simulink model "HvacCtrlrRe"  (R2022b)
settings: Solver=FixedStepDiscrete  StartTime=0.0  StopTime=10.0  FixedStep=0.2  RelTol=1e-3
settings Model: Creator=blue.ge  LastModifiedBy=blue.ge
settings DataIOCC: SaveFormat=StructureWithTime
settings OptimizationCC: DefaultParameterBehavior=Inlined  EnhancedBackFolding=on  PassReuseOutputArgsAs=Individual arguments  ZeroExternalMemoryAtStartup=off  ZeroInternalMemoryAtStartup=off  OptimizeBlockOrder=speed  DifferentSizesBufferReuse=on  OptimizationCustomize=off  EfficientTunableParamExpr=on
settings DebuggingCC: ParameterTunabilityLossMsg=error
settings ModelReferenceCC: ModelReferenceNumInstancesAllowed=Single
settings SFSimCC: MATLABDynamicMemAlloc=off
settings RTWCC: Description=AUTOSAR  SystemTargetFile=autosar.tlc  GenCodeOnly=on  TemplateMakefile=ert_default_tmf
settings CodeAppCC: ForceParamTrailComments=on  IgnoreCustomStorageClasses=off  OperatorAnnotations=on  SimulinkDataObjDesc=on  SFDataObjDesc=on  InsertBlockDesc=on
settings STFCustomTargetCC: TargetLangStandard=C99 (ISO)  MultiwordLength=256  ParMdlRefBuildCompliant=on  CompOptLevelCompliant=on  IncludeMdlTerminateFcn=off  CombineOutputUpdateFcns=on  SuppressErrorStatus=on  ERTCustomFileBanners=on  SupportNonFinite=off  SupportComplex=off  SupportContinuousTime=off  SupportNonInlinedSFcns=off  RemoveResetFunc=on  MATLABClassNameForMDSCustomization=Simulink.SoftwareTarget.ERTCustomization  AutosarCompliant=on  UseToolchainInfoCompliant=on  LookupTableObjectStructAxisOrder=2,1,3,4,...  LUTObjectStructOrderEvenSpacing=Size,Table,Breakpoints  SystemTargetFile=autosar.tlc
settings ERTTargetCC: TargetLangStandard=C99 (ISO)  ConcurrentExecutionCompliant=off  IncludeMdlTerminateFcn=off  SuppressErrorStatus=on  SupportNonFinite=off  SupportComplex=off  ModelStepFunctionPrototypeControlCompliant=off  CPPClassGenCompliant=off  AutosarCompliant=on  LookupTableObjectStructAxisOrder=2,1,3,4,...  LUTObjectStructOrderEvenSpacing=Size,Table,Breakpoints  InlinedParameterPlacement=NonHierarchical  ERTCustomFileTemplate=example_file_process.tlc

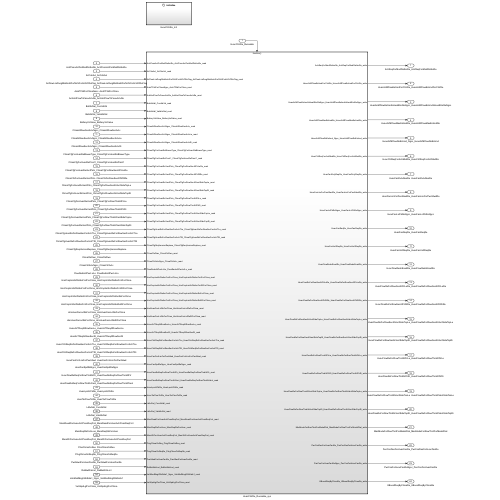
[thumbnail: Simulink's stored preview of the top-level diagram]
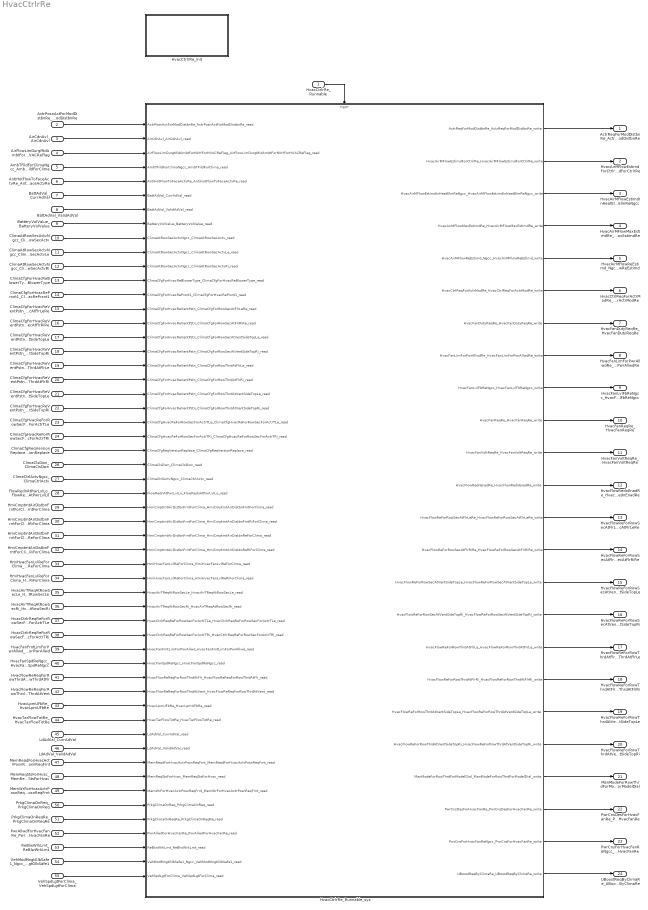
== system HvacCtrlrRe ==
  HvacCtrlrRe_Runnable : Inport  SampleTime=0.2  OutputFunctionCall=on
  ActrPosnActForModDistbnRe_ActrPosnActForModDistbnRe : Inport  Port=2  OutMin=0  OutMax=100  OutDataTypeStr=Perc9  PortDimensions=1
  AirCdnAvl_AirCdnAvl : Inport  Port=3  OutDataTypeStr=Boolean  PortDimensions=1
  AirFlowLimDurgMidAmbtForNVHForHVACReFlag_AirFlowLimDurgMidAmbtForNVHForHVACReFlag : Inport  Port=4  OutDataTypeStr=Boolean  PortDimensions=1
  AmbTFildForClimaNgcc_AmbTFildForClima : Inport  Port=5  OutDataTypeStr=T30  PortDimensions=1
  AntiHotFlowToFaceActvRe_AntiHotFlowToFaceActvRe : Inport  Port=6  OutDataTypeStr=Boolean  PortDimensions=1
  BattAdVal_CurrAdVal : Inport  Port=7  OutDataTypeStr=BatCurrAd  PortDimensions=1
  BattAdVal_ValidAdVal : Inport  Port=8  OutDataTypeStr=BatValidAd  PortDimensions=1
  BatteryVolValue_BatteryVolValue : Inport  Port=9  OutDataTypeStr=BatteryVolValue  PortDimensions=1
  ClimaAtRowSecActvNgcc_ClimaAtRowSecActv : Inport  Port=10  OutDataTypeStr=Boolean  PortDimensions=1
  ClimaAtRowSecActvNgcc_ClimaAtRowSecActvLe : Inport  Port=11  OutDataTypeStr=Boolean  PortDimensions=1
  ClimaAtRowSecActvNgcc_ClimaAtRowSecActvRi : Inport  Port=12  OutDataTypeStr=Boolean  PortDimensions=1
  ClimaCfgForHvacReBlowerType_ClimaCfgForHvacReBlowerType : Inport  Port=13  OutDataTypeStr=Enum: ClimaCfgForHvacReBlowerType  PortDimensions=1
  ClimaCfgForHvacRePrsnt1_ClimaCfgForHvacRePrsnt1 : Inport  Port=14  OutDataTypeStr=Enum: CarCfgForHvacRe  PortDimensions=1
  ClimaCfgForHvacReVentPstn_ClimaCfgForRowSecAtFlrLeRe : Inport  Port=15  OutDataTypeStr=UInt8  PortDimensions=1
  ClimaCfgForHvacReVentPstn_ClimaCfgForRowSecAtFlrRiRe : Inport  Port=16  OutDataTypeStr=UInt8  PortDimensions=1
  ClimaCfgForHvacReVentPstn_ClimaCfgForRowSecAtVentSideTopLe : Inport  Port=17  OutDataTypeStr=UInt8  PortDimensions=1
  ClimaCfgForHvacReVentPstn_ClimaCfgForRowSecAtVentSideTopRi : Inport  Port=18  OutDataTypeStr=UInt8  PortDimensions=1
  ClimaCfgForHvacReVentPstn_ClimaCfgForRowThrdAtFlrLe : Inport  Port=19  OutDataTypeStr=UInt8  PortDimensions=1
  ClimaCfgForHvacReVentPstn_ClimaCfgForRowThrdAtFlrRi : Inport  Port=20  OutDataTypeStr=UInt8  PortDimensions=1
  ClimaCfgForHvacReVentPstn_ClimaCfgForRowThrdAtVentSideTopLe : Inport  Port=21  OutDataTypeStr=UInt8  PortDimensions=1
  ClimaCfgForHvacReVentPstn_ClimaCfgForRowThrdAtVentSideTopRi : Inport  Port=22  OutDataTypeStr=UInt8  PortDimensions=1
  ClimaCfgHvacReForRowSecForActrTLe_ClimaCfgHvacReForRowSecForActrTLe : Inport  Port=23  OutDataTypeStr=UInt8  PortDimensions=1
  ClimaCfgHvacReForRowSecForActrTRi_ClimaCfgHvacReForRowSecForActrTRi : Inport  Port=24  OutDataTypeStr=UInt8  PortDimensions=1
  ClimaCfgReqVersionReplace_ClimaCfgReqVersionReplace : Inport  Port=25  OutDataTypeStr=UInt8  PortDimensions=1
  ClimaClsDwn_ClimaClsDwn : Inport  Port=26  OutDataTypeStr=Boolean  PortDimensions=1
  ClimaCtrlActvNgcc_ClimaCtrlActv : Inport  Port=27  OutDataTypeStr=Boolean  PortDimensions=1
  FlowRednAtPwrLvlLo_FlowRednAtPwrLvlLo : Inport  Port=28  OutDataTypeStr=Boolean  PortDimensions=1
  HmiCmptmtAirDistbnFrntForClima_HmiCmptmtAirDistbnFrntForClima : Inport  Port=29  OutDataTypeStr=Enum: HmiCmptmtAirDistbnFrnt  PortDimensions=1
  HmiCmptmtAirDistbnFrntForClima_HmiCmptmtAirDistbnFrntRiForClima : Inport  Port=30  OutDataTypeStr=Enum: HmiCmptmtAirDistbnFrnt  PortDimensions=1
  HmiCmptmtAirDistbnFrntForClima_HmiCmptmtAirDistbnReForClima : Inport  Port=31  OutDataTypeStr=Enum: HmiCmptmtAirDistbnFrnt  PortDimensions=1
  HmiCmptmtAirDistbnFrntForClima_HmiCmptmtAirDistbnReRiForClima : Inport  Port=32  OutDataTypeStr=Enum: HmiCmptmtAirDistbnFrnt  PortDimensions=1
  HmiHvacFanLvlReForClima_HmiHvacFanLvlReForClima : Inport  Port=33  OutDataTypeStr=Enum: HmiHvacFanLvl  PortDimensions=1
  HmiHvacFanLvlReForClima_HmiHvacFanLvlReRiForClima : Inport  Port=34  OutDataTypeStr=Enum: HmiHvacFanLvl  PortDimensions=1
  HvacAirTReqAtRowSecLe_HvacAirTReqAtRowSecLe : Inport  Port=35  OutDataTypeStr=T30  PortDimensions=1
  HvacAirTReqAtRowSecRi_HvacAirTReqAtRowSecRi : Inport  Port=36  OutDataTypeStr=T30  PortDimensions=1
  HvacCtrlrReqReForRowSecForActrTLe_HvacCtrlrReqReForRowSecForActrTLe : Inport  Port=37  OutDataTypeStr=Perc30  PortDimensions=1
  HvacCtrlrReqReForRowSecForActrTRi_HvacCtrlrReqReForRowSecForActrTRi : Inport  Port=38  OutDataTypeStr=Perc30  PortDimensions=1
  HvacFanFrntLimForPwrAllwd_HvacFanFrntLimForPwrAllwd : Inport  Port=39  OutDataTypeStr=Enum: HvacFanFrntLimForPwrAllwd  PortDimensions=1
  HvacFanSpdReNgcc_HvacFanSpdReNgcc : Inport  Port=40  OutDataTypeStr=HvacFanSpd  PortDimensions=1
  HvacFlowReReqForRowThrdAtFlr_HvacFlowReReqForRowThrdAtFlr : Inport  Port=41  OutDataTypeStr=AirFlow30  PortDimensions=1
  HvacFlowReReqForRowThrdAtVent_HvacFlowReReqForRowThrdAtVent : Inport  Port=42  OutDataTypeStr=AirFlow30  PortDimensions=1
  HvacLpmUFbRe_HvacLpmUFbRe : Inport  Port=43  OutMin=0  OutMax=100  OutDataTypeStr=Perc9  PortDimensions=1
  HvacTarFlowTotRe_HvacTarFlowTotRe : Inport  Port=44  OutDataTypeStr=AirFlow30  PortDimensions=1
  LdAdVal_CurrAdVal : Inport  Port=45  OutDataTypeStr=Bus: LdAd  PortDimensions=1  BusOutputAsStruct=on
  LdAdVal_ValidAdVal : Inport  Port=46  OutDataTypeStr=Bus: LdAd  PortDimensions=1  BusOutputAsStruct=on
  MemReadForHvacActrPosnReqFrnt_MemReadForHvacActrPosnReqFrnt : Inport  Port=47  OutDataTypeStr=Bus: MemRecForHvacActrPosnReqFrnt  PortDimensions=1  BusOutputAsStruct=on
  MemReqStsForHvac_MemReqStsForHvac : Inport  Port=48  OutDataTypeStr=Enum: MemReqSts  PortDimensions=1
  MemWrForHvacActrPosnReqFrnt_MemWrForHvacActrPosnReqFrnt : Inport  Port=49  OutDataTypeStr=Bus: MemRecForHvacActrPosnReqFrnt  PortDimensions=1  BusOutputAsStruct=on
  PrkgClimaOnReq_PrkgClimaOnReq : Inport  Port=50  OutDataTypeStr=Boolean  PortDimensions=1
  PrkgClimaOnReqRe_PrkgClimaOnReqRe : Inport  Port=51  OutDataTypeStr=Enum: OnOff1  PortDimensions=1
  PwrAllwdForHvacFanRe_PwrAllwdForHvacFanRe : Inport  Port=52  OutDataTypeStr=Pwr33  PortDimensions=1
  ReBlwWrkLmt_ReBlwWrkLmt : Inport  Port=53  OutDataTypeStr=UInt8  PortDimensions=1
  VehModMngtGlbSafe1_Ngcc_VehModMngtGlbSafe1 : Inport  Port=54  OutDataTypeStr=Bus: VehModMngtGlbSafe1  PortDimensions=1  BusOutputAsStruct=on
  VehSpdLgtForClima_VehSpdLgtForClima : Inport  Port=55  OutDataTypeStr=Bus: VehSpdLgtForClima  PortDimensions=1  BusOutputAsStruct=on
  HvacCtrlrRe_Init : Subsystem
  HvacCtrlrRe_Runnable_sys : Subsystem
  ActrReqForModDistbnRe_ActrReqForModDistbnRe : Outport  OutMin=0  OutMax=100  OutDataTypeStr=Perc9  PortDimensions=1
  HvacAirMFlowEstimdForCtrlrRe_HvacAirMFlowEstimdForCtrlrRe : Outport  Port=2  OutDataTypeStr=AirFlow30  PortDimensions=1
  HvacAirMFlowEstimdInHeatElmReNgcc_HvacAirMFlowEstimdInHeatElmReNgcc : Outport  Port=3  OutDataTypeStr=AirFlow30  PortDimensions=1
  HvacAirMFlowMaxEstimdRe_HvacAirMFlowMaxEstimdRe : Outport  Port=4  OutDataTypeStr=AirFlow30  PortDimensions=1
  HvacAirMFlowReEstimd_Ngcc_HvacAirMFlowReEstimd : Outport  Port=5  OutMin=0  OutMax=1000  OutDataTypeStr=MFlow1  PortDimensions=1
  HvacCtrlReqForActrModRe_HvacCtrlReqForActrModRe : Outport  Port=6  OutDataTypeStr=Perc30  PortDimensions=1
  HvacFanDutyReqRe_HvacFanDutyReqRe : Outport  Port=7  OutMin=0  OutMax=100  OutDataTypeStr=Perc9  PortDimensions=1
  HvacFanLimForPwrAllwdRe_HvacFanLimForPwrAllwdRe : Outport  Port=8  OutDataTypeStr=Enum: HvacFanFrntLimForPwrAllwd  PortDimensions=1
  HvacFanLvlFbReNgcc_HvacFanLvlFbReNgcc : Outport  Port=9  OutDataTypeStr=Enum: HmiHvacFanLvl  PortDimensions=1
  HvacFanReqRe_HvacFanReqRe : Outport  Port=10  OutDataTypeStr=HvacFanSpd  PortDimensions=1
  HvacFanVoltReqRe_HvacFanVoltReqRe : Outport  Port=11  OutMin=0  OutMax=100  OutDataTypeStr=Perc9  PortDimensions=1
  HvacFlowRednEnadRe_HvacFlowRednEnadRe : Outport  Port=12  OutDataTypeStr=Boolean  PortDimensions=1
  HvacFlowReForRowSecAtFlrLeRe_HvacFlowReForRowSecAtFlrLeRe : Outport  Port=13  OutDataTypeStr=AirFlow30  PortDimensions=1
  HvacFlowReForRowSecAtFlrRiRe_HvacFlowReForRowSecAtFlrRiRe : Outport  Port=14  OutDataTypeStr=AirFlow30  PortDimensions=1
  HvacFlowReForRowSecAtVentSideTopLe_HvacFlowReForRowSecAtVentSideTopLe : Outport  Port=15  OutDataTypeStr=AirFlow30  PortDimensions=1
  HvacFlowReForRowSecAtVentSideTopRi_HvacFlowReForRowSecAtVentSideTopRi : Outport  Port=16  OutDataTypeStr=AirFlow30  PortDimensions=1
  HvacFlowReForRowThrdAtFlrLe_HvacFlowReForRowThrdAtFlrLe : Outport  Port=17  OutDataTypeStr=AirFlow30  PortDimensions=1
  HvacFlowReForRowThrdAtFlrRi_HvacFlowReForRowThrdAtFlrRi : Outport  Port=18  OutDataTypeStr=AirFlow30  PortDimensions=1
  HvacFlowReForRowThrdAtVentSideTopLe_HvacFlowReForRowThrdAtVentSideTopLe : Outport  Port=19  OutDataTypeStr=AirFlow30  PortDimensions=1
  HvacFlowReForRowThrdAtVentSideTopRi_HvacFlowReForRowThrdAtVentSideTopRi : Outport  Port=20  OutDataTypeStr=AirFlow30  PortDimensions=1
  ManModeForRowThrdForModelDial_ManModeForRowThrdForModelDial : Outport  Port=21  OutDataTypeStr=Enum: HmiCmptmtAirDistbnFrnt  PortDimensions=1
  PwrCnsDesForHvacFanRe_PwrCnsDesForHvacFanRe : Outport  Port=22  OutDataTypeStr=Pwr33  PortDimensions=1
  PwrCnsForHvacFanReNgcc_PwrCnsForHvacFanRe : Outport  Port=23  OutDataTypeStr=Pwr33  PortDimensions=1
  UBoostReqByClimaRe_UBoostReqByClimaRe : Outport  Port=24  OutDataTypeStr=Enum: ReqSts1  PortDimensions=1
  wires:
  ActrPosnActForModDistbnRe_ActrPosnActForModDistbnRe/1 -> HvacCtrlrRe_Runnable_sys/1(ActrPosnActForModDistbnRe_ActrPosnActForModDistbnRe_read)
  AirCdnAvl_AirCdnAvl/1 -> HvacCtrlrRe_Runnable_sys/2(AirCdnAvl_AirCdnAvl_read)
  AirFlowLimDurgMidAmbtForNVHForHVACReFlag_AirFlowLimDurgMidAmbtForNVHForHVACReFlag/1 -> HvacCtrlrRe_Runnable_sys/3(AirFlowLimDurgMidAmbtForNVHForHVACReFlag_AirFlowLimDurgMidAmbtForNVHForHVACReFlag_read)
  AmbTFildForClimaNgcc_AmbTFildForClima/1 -> HvacCtrlrRe_Runnable_sys/4(AmbTFildForClimaNgcc_AmbTFildForClima_read)
  AntiHotFlowToFaceActvRe_AntiHotFlowToFaceActvRe/1 -> HvacCtrlrRe_Runnable_sys/5(AntiHotFlowToFaceActvRe_AntiHotFlowToFaceActvRe_read)
  BattAdVal_CurrAdVal/1 -> HvacCtrlrRe_Runnable_sys/6(BattAdVal_CurrAdVal_read)
  BattAdVal_ValidAdVal/1 -> HvacCtrlrRe_Runnable_sys/7(BattAdVal_ValidAdVal_read)
  BatteryVolValue_BatteryVolValue/1 -> HvacCtrlrRe_Runnable_sys/8(BatteryVolValue_BatteryVolValue_read)
  ClimaAtRowSecActvNgcc_ClimaAtRowSecActv/1 -> HvacCtrlrRe_Runnable_sys/9(ClimaAtRowSecActvNgcc_ClimaAtRowSecActv_read)
  ClimaAtRowSecActvNgcc_ClimaAtRowSecActvLe/1 -> HvacCtrlrRe_Runnable_sys/10(ClimaAtRowSecActvNgcc_ClimaAtRowSecActvLe_read)
  ClimaAtRowSecActvNgcc_ClimaAtRowSecActvRi/1 -> HvacCtrlrRe_Runnable_sys/11(ClimaAtRowSecActvNgcc_ClimaAtRowSecActvRi_read)
  ClimaCfgForHvacReBlowerType_ClimaCfgForHvacReBlowerType/1 -> HvacCtrlrRe_Runnable_sys/12(ClimaCfgForHvacReBlowerType_ClimaCfgForHvacReBlowerType_read)
  ClimaCfgForHvacRePrsnt1_ClimaCfgForHvacRePrsnt1/1 -> HvacCtrlrRe_Runnable_sys/13(ClimaCfgForHvacRePrsnt1_ClimaCfgForHvacRePrsnt1_read)
  ClimaCfgForHvacReVentPstn_ClimaCfgForRowSecAtFlrLeRe/1 -> HvacCtrlrRe_Runnable_sys/14(ClimaCfgForHvacReVentPstn_ClimaCfgForRowSecAtFlrLeRe_read)
  ClimaCfgForHvacReVentPstn_ClimaCfgForRowSecAtFlrRiRe/1 -> HvacCtrlrRe_Runnable_sys/15(ClimaCfgForHvacReVentPstn_ClimaCfgForRowSecAtFlrRiRe_read)
  ClimaCfgForHvacReVentPstn_ClimaCfgForRowSecAtVentSideTopLe/1 -> HvacCtrlrRe_Runnable_sys/16(ClimaCfgForHvacReVentPstn_ClimaCfgForRowSecAtVentSideTopLe_read)
  ClimaCfgForHvacReVentPstn_ClimaCfgForRowSecAtVentSideTopRi/1 -> HvacCtrlrRe_Runnable_sys/17(ClimaCfgForHvacReVentPstn_ClimaCfgForRowSecAtVentSideTopRi_read)
  ClimaCfgForHvacReVentPstn_ClimaCfgForRowThrdAtFlrLe/1 -> HvacCtrlrRe_Runnable_sys/18(ClimaCfgForHvacReVentPstn_ClimaCfgForRowThrdAtFlrLe_read)
  ClimaCfgForHvacReVentPstn_ClimaCfgForRowThrdAtFlrRi/1 -> HvacCtrlrRe_Runnable_sys/19(ClimaCfgForHvacReVentPstn_ClimaCfgForRowThrdAtFlrRi_read)
  ClimaCfgForHvacReVentPstn_ClimaCfgForRowThrdAtVentSideTopLe/1 -> HvacCtrlrRe_Runnable_sys/20(ClimaCfgForHvacReVentPstn_ClimaCfgForRowThrdAtVentSideTopLe_read)
  ClimaCfgForHvacReVentPstn_ClimaCfgForRowThrdAtVentSideTopRi/1 -> HvacCtrlrRe_Runnable_sys/21(ClimaCfgForHvacReVentPstn_ClimaCfgForRowThrdAtVentSideTopRi_read)
  ClimaCfgHvacReForRowSecForActrTLe_ClimaCfgHvacReForRowSecForActrTLe/1 -> HvacCtrlrRe_Runnable_sys/22(ClimaCfgHvacReForRowSecForActrTLe_ClimaCfgHvacReForRowSecForActrTLe_read)
  ClimaCfgHvacReForRowSecForActrTRi_ClimaCfgHvacReForRowSecForActrTRi/1 -> HvacCtrlrRe_Runnable_sys/23(ClimaCfgHvacReForRowSecForActrTRi_ClimaCfgHvacReForRowSecForActrTRi_read)
  ClimaCfgReqVersionReplace_ClimaCfgReqVersionReplace/1 -> HvacCtrlrRe_Runnable_sys/24(ClimaCfgReqVersionReplace_ClimaCfgReqVersionReplace_read)
  ClimaClsDwn_ClimaClsDwn/1 -> HvacCtrlrRe_Runnable_sys/25(ClimaClsDwn_ClimaClsDwn_read)
  ClimaCtrlActvNgcc_ClimaCtrlActv/1 -> HvacCtrlrRe_Runnable_sys/26(ClimaCtrlActvNgcc_ClimaCtrlActv_read)
  FlowRednAtPwrLvlLo_FlowRednAtPwrLvlLo/1 -> HvacCtrlrRe_Runnable_sys/27(FlowRednAtPwrLvlLo_FlowRednAtPwrLvlLo_read)
  HmiCmptmtAirDistbnFrntForClima_HmiCmptmtAirDistbnFrntForClima/1 -> HvacCtrlrRe_Runnable_sys/28(HmiCmptmtAirDistbnFrntForClima_HmiCmptmtAirDistbnFrntForClima_read)
  HmiCmptmtAirDistbnFrntForClima_HmiCmptmtAirDistbnFrntRiForClima/1 -> HvacCtrlrRe_Runnable_sys/29(HmiCmptmtAirDistbnFrntForClima_HmiCmptmtAirDistbnFrntRiForClima_read)
  HmiCmptmtAirDistbnFrntForClima_HmiCmptmtAirDistbnReForClima/1 -> HvacCtrlrRe_Runnable_sys/30(HmiCmptmtAirDistbnFrntForClima_HmiCmptmtAirDistbnReForClima_read)
  HmiCmptmtAirDistbnFrntForClima_HmiCmptmtAirDistbnReRiForClima/1 -> HvacCtrlrRe_Runnable_sys/31(HmiCmptmtAirDistbnFrntForClima_HmiCmptmtAirDistbnReRiForClima_read)
  HmiHvacFanLvlReForClima_HmiHvacFanLvlReForClima/1 -> HvacCtrlrRe_Runnable_sys/32(HmiHvacFanLvlReForClima_HmiHvacFanLvlReForClima_read)
  HmiHvacFanLvlReForClima_HmiHvacFanLvlReRiForClima/1 -> HvacCtrlrRe_Runnable_sys/33(HmiHvacFanLvlReForClima_HmiHvacFanLvlReRiForClima_read)
  HvacAirTReqAtRowSecLe_HvacAirTReqAtRowSecLe/1 -> HvacCtrlrRe_Runnable_sys/34(HvacAirTReqAtRowSecLe_HvacAirTReqAtRowSecLe_read)
  HvacAirTReqAtRowSecRi_HvacAirTReqAtRowSecRi/1 -> HvacCtrlrRe_Runnable_sys/35(HvacAirTReqAtRowSecRi_HvacAirTReqAtRowSecRi_read)
  HvacCtrlrReqReForRowSecForActrTLe_HvacCtrlrReqReForRowSecForActrTLe/1 -> HvacCtrlrRe_Runnable_sys/36(HvacCtrlrReqReForRowSecForActrTLe_HvacCtrlrReqReForRowSecForActrTLe_read)
  HvacCtrlrReqReForRowSecForActrTRi_HvacCtrlrReqReForRowSecForActrTRi/1 -> HvacCtrlrRe_Runnable_sys/37(HvacCtrlrReqReForRowSecForActrTRi_HvacCtrlrReqReForRowSecForActrTRi_read)
  HvacFanFrntLimForPwrAllwd_HvacFanFrntLimForPwrAllwd/1 -> HvacCtrlrRe_Runnable_sys/38(HvacFanFrntLimForPwrAllwd_HvacFanFrntLimForPwrAllwd_read)
  HvacFanSpdReNgcc_HvacFanSpdReNgcc/1 -> HvacCtrlrRe_Runnable_sys/39(HvacFanSpdReNgcc_HvacFanSpdReNgcc_read)
  HvacFlowReReqForRowThrdAtFlr_HvacFlowReReqForRowThrdAtFlr/1 -> HvacCtrlrRe_Runnable_sys/40(HvacFlowReReqForRowThrdAtFlr_HvacFlowReReqForRowThrdAtFlr_read)
  HvacFlowReReqForRowThrdAtVent_HvacFlowReReqForRowThrdAtVent/1 -> HvacCtrlrRe_Runnable_sys/41(HvacFlowReReqForRowThrdAtVent_HvacFlowReReqForRowThrdAtVent_read)
  HvacLpmUFbRe_HvacLpmUFbRe/1 -> HvacCtrlrRe_Runnable_sys/42(HvacLpmUFbRe_HvacLpmUFbRe_read)
  HvacTarFlowTotRe_HvacTarFlowTotRe/1 -> HvacCtrlrRe_Runnable_sys/43(HvacTarFlowTotRe_HvacTarFlowTotRe_read)
  LdAdVal_CurrAdVal/1 -> HvacCtrlrRe_Runnable_sys/44(LdAdVal_CurrAdVal_read)
  LdAdVal_ValidAdVal/1 -> HvacCtrlrRe_Runnable_sys/45(LdAdVal_ValidAdVal_read)
  MemReadForHvacActrPosnReqFrnt_MemReadForHvacActrPosnReqFrnt/1 -> HvacCtrlrRe_Runnable_sys/46(MemReadForHvacActrPosnReqFrnt_MemReadForHvacActrPosnReqFrnt_read)
  MemReqStsForHvac_MemReqStsForHvac/1 -> HvacCtrlrRe_Runnable_sys/47(MemReqStsForHvac_MemReqStsForHvac_read)
  MemWrForHvacActrPosnReqFrnt_MemWrForHvacActrPosnReqFrnt/1 -> HvacCtrlrRe_Runnable_sys/48(MemWrForHvacActrPosnReqFrnt_MemWrForHvacActrPosnReqFrnt_read)
  PrkgClimaOnReq_PrkgClimaOnReq/1 -> HvacCtrlrRe_Runnable_sys/49(PrkgClimaOnReq_PrkgClimaOnReq_read)
  PrkgClimaOnReqRe_PrkgClimaOnReqRe/1 -> HvacCtrlrRe_Runnable_sys/50(PrkgClimaOnReqRe_PrkgClimaOnReqRe_read)
  PwrAllwdForHvacFanRe_PwrAllwdForHvacFanRe/1 -> HvacCtrlrRe_Runnable_sys/51(PwrAllwdForHvacFanRe_PwrAllwdForHvacFanRe_read)
  ReBlwWrkLmt_ReBlwWrkLmt/1 -> HvacCtrlrRe_Runnable_sys/52(ReBlwWrkLmt_ReBlwWrkLmt_read)
  VehModMngtGlbSafe1_Ngcc_VehModMngtGlbSafe1/1 -> HvacCtrlrRe_Runnable_sys/53(VehModMngtGlbSafe1_Ngcc_VehModMngtGlbSafe1_read)
  VehSpdLgtForClima_VehSpdLgtForClima/1 -> HvacCtrlrRe_Runnable_sys/54(VehSpdLgtForClima_VehSpdLgtForClima_read)
  HvacCtrlrRe_Runnable_sys/1(ActrReqForModDistbnRe_ActrReqForModDistbnRe_write) -> ActrReqForModDistbnRe_ActrReqForModDistbnRe/1
  HvacCtrlrRe_Runnable_sys/2(HvacAirMFlowEstimdForCtrlrRe_HvacAirMFlowEstimdForCtrlrRe_write) -> HvacAirMFlowEstimdForCtrlrRe_HvacAirMFlowEstimdForCtrlrRe/1
  HvacCtrlrRe_Runnable_sys/3(HvacAirMFlowEstimdInHeatElmReNgcc_HvacAirMFlowEstimdInHeatElmReNgcc_write) -> HvacAirMFlowEstimdInHeatElmReNgcc_HvacAirMFlowEstimdInHeatElmReNgcc/1
  HvacCtrlrRe_Runnable_sys/4(HvacAirMFlowMaxEstimdRe_HvacAirMFlowMaxEstimdRe_write) -> HvacAirMFlowMaxEstimdRe_HvacAirMFlowMaxEstimdRe/1
  HvacCtrlrRe_Runnable_sys/5(HvacAirMFlowReEstimd_Ngcc_HvacAirMFlowReEstimd_write) -> HvacAirMFlowReEstimd_Ngcc_HvacAirMFlowReEstimd/1
  HvacCtrlrRe_Runnable_sys/6(HvacCtrlReqForActrModRe_HvacCtrlReqForActrModRe_write) -> HvacCtrlReqForActrModRe_HvacCtrlReqForActrModRe/1
  HvacCtrlrRe_Runnable_sys/7(HvacFanDutyReqRe_HvacFanDutyReqRe_write) -> HvacFanDutyReqRe_HvacFanDutyReqRe/1
  HvacCtrlrRe_Runnable_sys/8(HvacFanLimForPwrAllwdRe_HvacFanLimForPwrAllwdRe_write) -> HvacFanLimForPwrAllwdRe_HvacFanLimForPwrAllwdRe/1
  HvacCtrlrRe_Runnable_sys/9(HvacFanLvlFbReNgcc_HvacFanLvlFbReNgcc_write) -> HvacFanLvlFbReNgcc_HvacFanLvlFbReNgcc/1
  HvacCtrlrRe_Runnable_sys/10(HvacFanReqRe_HvacFanReqRe_write) -> HvacFanReqRe_HvacFanReqRe/1
  HvacCtrlrRe_Runnable_sys/11(HvacFanVoltReqRe_HvacFanVoltReqRe_write) -> HvacFanVoltReqRe_HvacFanVoltReqRe/1
  HvacCtrlrRe_Runnable_sys/12(HvacFlowRednEnadRe_HvacFlowRednEnadRe_write) -> HvacFlowRednEnadRe_HvacFlowRednEnadRe/1
  HvacCtrlrRe_Runnable_sys/13(HvacFlowReForRowSecAtFlrLeRe_HvacFlowReForRowSecAtFlrLeRe_write) -> HvacFlowReForRowSecAtFlrLeRe_HvacFlowReForRowSecAtFlrLeRe/1
  HvacCtrlrRe_Runnable_sys/14(HvacFlowReForRowSecAtFlrRiRe_HvacFlowReForRowSecAtFlrRiRe_write) -> HvacFlowReForRowSecAtFlrRiRe_HvacFlowReForRowSecAtFlrRiRe/1
  HvacCtrlrRe_Runnable_sys/15(HvacFlowReForRowSecAtVentSideTopLe_HvacFlowReForRowSecAtVentSideTopLe_write) -> HvacFlowReForRowSecAtVentSideTopLe_HvacFlowReForRowSecAtVentSideTopLe/1
  HvacCtrlrRe_Runnable_sys/16(HvacFlowReForRowSecAtVentSideTopRi_HvacFlowReForRowSecAtVentSideTopRi_write) -> HvacFlowReForRowSecAtVentSideTopRi_HvacFlowReForRowSecAtVentSideTopRi/1
  HvacCtrlrRe_Runnable_sys/17(HvacFlowReForRowThrdAtFlrLe_HvacFlowReForRowThrdAtFlrLe_write) -> HvacFlowReForRowThrdAtFlrLe_HvacFlowReForRowThrdAtFlrLe/1
  HvacCtrlrRe_Runnable_sys/18(HvacFlowReForRowThrdAtFlrRi_HvacFlowReForRowThrdAtFlrRi_write) -> HvacFlowReForRowThrdAtFlrRi_HvacFlowReForRowThrdAtFlrRi/1
  HvacCtrlrRe_Runnable_sys/19(HvacFlowReForRowThrdAtVentSideTopLe_HvacFlowReForRowThrdAtVentSideTopLe_write) -> HvacFlowReForRowThrdAtVentSideTopLe_HvacFlowReForRowThrdAtVentSideTopLe/1
  HvacCtrlrRe_Runnable_sys/20(HvacFlowReForRowThrdAtVentSideTopRi_HvacFlowReForRowThrdAtVentSideTopRi_write) -> HvacFlowReForRowThrdAtVentSideTopRi_HvacFlowReForRowThrdAtVentSideTopRi/1
  HvacCtrlrRe_Runnable_sys/21(ManModeForRowThrdForModelDial_ManModeForRowThrdForModelDial_write) -> ManModeForRowThrdForModelDial_ManModeForRowThrdForModelDial/1
  HvacCtrlrRe_Runnable_sys/22(PwrCnsDesForHvacFanRe_PwrCnsDesForHvacFanRe_write) -> PwrCnsDesForHvacFanRe_PwrCnsDesForHvacFanRe/1
  HvacCtrlrRe_Runnable_sys/23(PwrCnsForHvacFanReNgcc_PwrCnsForHvacFanRe_write) -> PwrCnsForHvacFanReNgcc_PwrCnsForHvacFanRe/1
  HvacCtrlrRe_Runnable_sys/24(UBoostReqByClimaRe_UBoostReqByClimaRe_write) -> UBoostReqByClimaRe_UBoostReqByClimaRe/1
  HvacCtrlrRe_Runnable/1 -> HvacCtrlrRe_Runnable_sys/Trigger

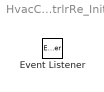
== system HvacCtrlrRe/HvacCtrlrRe_Init ==
  Event Listener : EventListener  EventName=reset

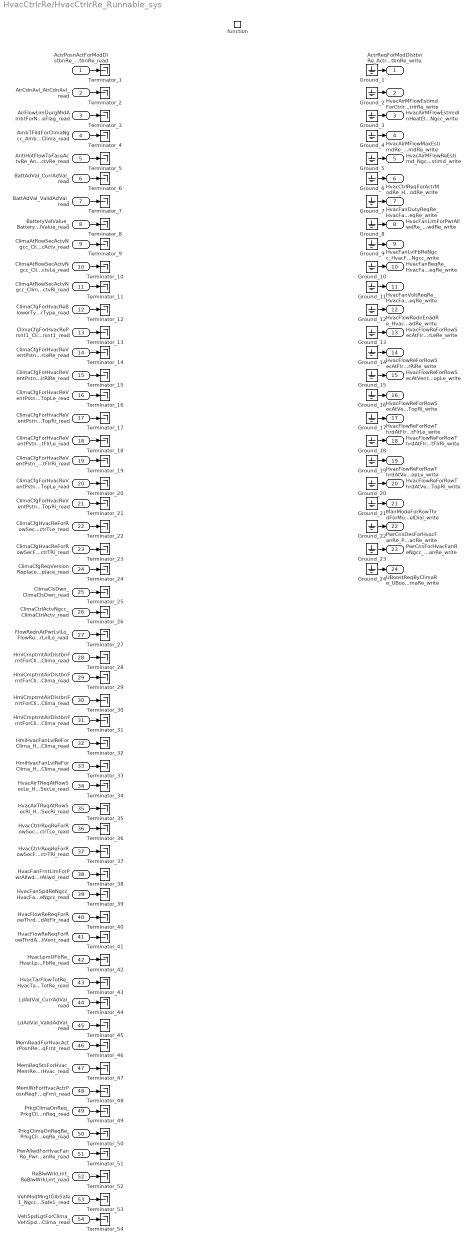
== system HvacCtrlrRe/HvacCtrlrRe_Runnable_sys ==
  ActrPosnActForModDistbnRe_ActrPosnActForModDistbnRe_read : Inport
  AirCdnAvl_AirCdnAvl_read : Inport  Port=2
  AirFlowLimDurgMidAmbtForNVHForHVACReFlag_AirFlowLimDurgMidAmbtForNVHForHVACReFlag_read : Inport  Port=3
  AmbTFildForClimaNgcc_AmbTFildForClima_read : Inport  Port=4
  AntiHotFlowToFaceActvRe_AntiHotFlowToFaceActvRe_read : Inport  Port=5
  BattAdVal_CurrAdVal_read : Inport  Port=6
  BattAdVal_ValidAdVal_read : Inport  Port=7
  BatteryVolValue_BatteryVolValue_read : Inport  Port=8
  ClimaAtRowSecActvNgcc_ClimaAtRowSecActv_read : Inport  Port=9
  ClimaAtRowSecActvNgcc_ClimaAtRowSecActvLe_read : Inport  Port=10
  ClimaAtRowSecActvNgcc_ClimaAtRowSecActvRi_read : Inport  Port=11
  ClimaCfgForHvacReBlowerType_ClimaCfgForHvacReBlowerType_read : Inport  Port=12
  ClimaCfgForHvacRePrsnt1_ClimaCfgForHvacRePrsnt1_read : Inport  Port=13
  ClimaCfgForHvacReVentPstn_ClimaCfgForRowSecAtFlrLeRe_read : Inport  Port=14
  ClimaCfgForHvacReVentPstn_ClimaCfgForRowSecAtFlrRiRe_read : Inport  Port=15
  ClimaCfgForHvacReVentPstn_ClimaCfgForRowSecAtVentSideTopLe_read : Inport  Port=16
  ClimaCfgForHvacReVentPstn_ClimaCfgForRowSecAtVentSideTopRi_read : Inport  Port=17
  ClimaCfgForHvacReVentPstn_ClimaCfgForRowThrdAtFlrLe_read : Inport  Port=18
  ClimaCfgForHvacReVentPstn_ClimaCfgForRowThrdAtFlrRi_read : Inport  Port=19
  ClimaCfgForHvacReVentPstn_ClimaCfgForRowThrdAtVentSideTopLe_read : Inport  Port=20
  ClimaCfgForHvacReVentPstn_ClimaCfgForRowThrdAtVentSideTopRi_read : Inport  Port=21
  ClimaCfgHvacReForRowSecForActrTLe_ClimaCfgHvacReForRowSecForActrTLe_read : Inport  Port=22
  ClimaCfgHvacReForRowSecForActrTRi_ClimaCfgHvacReForRowSecForActrTRi_read : Inport  Port=23
  ClimaCfgReqVersionReplace_ClimaCfgReqVersionReplace_read : Inport  Port=24
  ClimaClsDwn_ClimaClsDwn_read : Inport  Port=25
  ClimaCtrlActvNgcc_ClimaCtrlActv_read : Inport  Port=26
  FlowRednAtPwrLvlLo_FlowRednAtPwrLvlLo_read : Inport  Port=27
  HmiCmptmtAirDistbnFrntForClima_HmiCmptmtAirDistbnFrntForClima_read : Inport  Port=28
  HmiCmptmtAirDistbnFrntForClima_HmiCmptmtAirDistbnFrntRiForClima_read : Inport  Port=29
  HmiCmptmtAirDistbnFrntForClima_HmiCmptmtAirDistbnReForClima_read : Inport  Port=30
  HmiCmptmtAirDistbnFrntForClima_HmiCmptmtAirDistbnReRiForClima_read : Inport  Port=31
  HmiHvacFanLvlReForClima_HmiHvacFanLvlReForClima_read : Inport  Port=32
  HmiHvacFanLvlReForClima_HmiHvacFanLvlReRiForClima_read : Inport  Port=33
  HvacAirTReqAtRowSecLe_HvacAirTReqAtRowSecLe_read : Inport  Port=34
  HvacAirTReqAtRowSecRi_HvacAirTReqAtRowSecRi_read : Inport  Port=35
  HvacCtrlrReqReForRowSecForActrTLe_HvacCtrlrReqReForRowSecForActrTLe_read : Inport  Port=36
  HvacCtrlrReqReForRowSecForActrTRi_HvacCtrlrReqReForRowSecForActrTRi_read : Inport  Port=37
  HvacFanFrntLimForPwrAllwd_HvacFanFrntLimForPwrAllwd_read : Inport  Port=38
  HvacFanSpdReNgcc_HvacFanSpdReNgcc_read : Inport  Port=39
  HvacFlowReReqForRowThrdAtFlr_HvacFlowReReqForRowThrdAtFlr_read : Inport  Port=40
  HvacFlowReReqForRowThrdAtVent_HvacFlowReReqForRowThrdAtVent_read : Inport  Port=41
  HvacLpmUFbRe_HvacLpmUFbRe_read : Inport  Port=42
  HvacTarFlowTotRe_HvacTarFlowTotRe_read : Inport  Port=43
  LdAdVal_CurrAdVal_read : Inport  Port=44
  LdAdVal_ValidAdVal_read : Inport  Port=45
  MemReadForHvacActrPosnReqFrnt_MemReadForHvacActrPosnReqFrnt_read : Inport  Port=46
  MemReqStsForHvac_MemReqStsForHvac_read : Inport  Port=47
  MemWrForHvacActrPosnReqFrnt_MemWrForHvacActrPosnReqFrnt_read : Inport  Port=48
  PrkgClimaOnReq_PrkgClimaOnReq_read : Inport  Port=49
  PrkgClimaOnReqRe_PrkgClimaOnReqRe_read : Inport  Port=50
  PwrAllwdForHvacFanRe_PwrAllwdForHvacFanRe_read : Inport  Port=51
  ReBlwWrkLmt_ReBlwWrkLmt_read : Inport  Port=52
  VehModMngtGlbSafe1_Ngcc_VehModMngtGlbSafe1_read : Inport  Port=53
  VehSpdLgtForClima_VehSpdLgtForClima_read : Inport  Port=54
  function : TriggerPort  TriggerType=function-call  VariantControl=(inherit)  SampleTimeType=periodic  SampleTime=-1
  Ground_{1..24} (24 alike) : Ground
  Terminator_{1..54} (54 alike) : Terminator
  ActrReqForModDistbnRe_ActrReqForModDistbnRe_write : Outport
      settings: EnsureOutportIsVirtual=on
  HvacAirMFlowEstimdForCtrlrRe_HvacAirMFlowEstimdForCtrlrRe_write : Outport  Port=2
      settings: EnsureOutportIsVirtual=on
  HvacAirMFlowEstimdInHeatElmReNgcc_HvacAirMFlowEstimdInHeatElmReNgcc_write : Outport  Port=3
      settings: EnsureOutportIsVirtual=on
  HvacAirMFlowMaxEstimdRe_HvacAirMFlowMaxEstimdRe_write : Outport  Port=4
      settings: EnsureOutportIsVirtual=on
  HvacAirMFlowReEstimd_Ngcc_HvacAirMFlowReEstimd_write : Outport  Port=5
      settings: EnsureOutportIsVirtual=on
  HvacCtrlReqForActrModRe_HvacCtrlReqForActrModRe_write : Outport  Port=6
      settings: EnsureOutportIsVirtual=on
  HvacFanDutyReqRe_HvacFanDutyReqRe_write : Outport  Port=7
      settings: EnsureOutportIsVirtual=on
  HvacFanLimForPwrAllwdRe_HvacFanLimForPwrAllwdRe_write : Outport  Port=8
      settings: EnsureOutportIsVirtual=on
  HvacFanLvlFbReNgcc_HvacFanLvlFbReNgcc_write : Outport  Port=9
      settings: EnsureOutportIsVirtual=on
  HvacFanReqRe_HvacFanReqRe_write : Outport  Port=10
      settings: EnsureOutportIsVirtual=on
  HvacFanVoltReqRe_HvacFanVoltReqRe_write : Outport  Port=11
      settings: EnsureOutportIsVirtual=on
  HvacFlowRednEnadRe_HvacFlowRednEnadRe_write : Outport  Port=12
      settings: EnsureOutportIsVirtual=on
  HvacFlowReForRowSecAtFlrLeRe_HvacFlowReForRowSecAtFlrLeRe_write : Outport  Port=13
      settings: EnsureOutportIsVirtual=on
  HvacFlowReForRowSecAtFlrRiRe_HvacFlowReForRowSecAtFlrRiRe_write : Outport  Port=14
      settings: EnsureOutportIsVirtual=on
  HvacFlowReForRowSecAtVentSideTopLe_HvacFlowReForRowSecAtVentSideTopLe_write : Outport  Port=15
      settings: EnsureOutportIsVirtual=on
  HvacFlowReForRowSecAtVentSideTopRi_HvacFlowReForRowSecAtVentSideTopRi_write : Outport  Port=16
      settings: EnsureOutportIsVirtual=on
  HvacFlowReForRowThrdAtFlrLe_HvacFlowReForRowThrdAtFlrLe_write : Outport  Port=17
      settings: EnsureOutportIsVirtual=on
  HvacFlowReForRowThrdAtFlrRi_HvacFlowReForRowThrdAtFlrRi_write : Outport  Port=18
      settings: EnsureOutportIsVirtual=on
  HvacFlowReForRowThrdAtVentSideTopLe_HvacFlowReForRowThrdAtVentSideTopLe_write : Outport  Port=19
      settings: EnsureOutportIsVirtual=on
  HvacFlowReForRowThrdAtVentSideTopRi_HvacFlowReForRowThrdAtVentSideTopRi_write : Outport  Port=20
      settings: EnsureOutportIsVirtual=on
  ManModeForRowThrdForModelDial_ManModeForRowThrdForModelDial_write : Outport  Port=21
      settings: EnsureOutportIsVirtual=on
  PwrCnsDesForHvacFanRe_PwrCnsDesForHvacFanRe_write : Outport  Port=22
      settings: EnsureOutportIsVirtual=on
  PwrCnsForHvacFanReNgcc_PwrCnsForHvacFanRe_write : Outport  Port=23
      settings: EnsureOutportIsVirtual=on
  UBoostReqByClimaRe_UBoostReqByClimaRe_write : Outport  Port=24
      settings: EnsureOutportIsVirtual=on
  wires:
  ActrPosnActForModDistbnRe_ActrPosnActForModDistbnRe_read/1 -> Terminator_1/1
  AirCdnAvl_AirCdnAvl_read/1 -> Terminator_2/1
  AirFlowLimDurgMidAmbtForNVHForHVACReFlag_AirFlowLimDurgMidAmbtForNVHForHVACReFlag_read/1 -> Terminator_3/1
  AmbTFildForClimaNgcc_AmbTFildForClima_read/1 -> Terminator_4/1
  AntiHotFlowToFaceActvRe_AntiHotFlowToFaceActvRe_read/1 -> Terminator_5/1
  BattAdVal_CurrAdVal_read/1 -> Terminator_6/1
  BattAdVal_ValidAdVal_read/1 -> Terminator_7/1
  BatteryVolValue_BatteryVolValue_read/1 -> Terminator_8/1
  ClimaAtRowSecActvNgcc_ClimaAtRowSecActv_read/1 -> Terminator_9/1
  ClimaAtRowSecActvNgcc_ClimaAtRowSecActvLe_read/1 -> Terminator_10/1
  ClimaAtRowSecActvNgcc_ClimaAtRowSecActvRi_read/1 -> Terminator_11/1
  ClimaCfgForHvacReBlowerType_ClimaCfgForHvacReBlowerType_read/1 -> Terminator_12/1
  ClimaCfgForHvacRePrsnt1_ClimaCfgForHvacRePrsnt1_read/1 -> Terminator_13/1
  ClimaCfgForHvacReVentPstn_ClimaCfgForRowSecAtFlrLeRe_read/1 -> Terminator_14/1
  ClimaCfgForHvacReVentPstn_ClimaCfgForRowSecAtFlrRiRe_read/1 -> Terminator_15/1
  ClimaCfgForHvacReVentPstn_ClimaCfgForRowSecAtVentSideTopLe_read/1 -> Terminator_16/1
  ClimaCfgForHvacReVentPstn_ClimaCfgForRowSecAtVentSideTopRi_read/1 -> Terminator_17/1
  ClimaCfgForHvacReVentPstn_ClimaCfgForRowThrdAtFlrLe_read/1 -> Terminator_18/1
  ClimaCfgForHvacReVentPstn_ClimaCfgForRowThrdAtFlrRi_read/1 -> Terminator_19/1
  ClimaCfgForHvacReVentPstn_ClimaCfgForRowThrdAtVentSideTopLe_read/1 -> Terminator_20/1
  ClimaCfgForHvacReVentPstn_ClimaCfgForRowThrdAtVentSideTopRi_read/1 -> Terminator_21/1
  ClimaCfgHvacReForRowSecForActrTLe_ClimaCfgHvacReForRowSecForActrTLe_read/1 -> Terminator_22/1
  ClimaCfgHvacReForRowSecForActrTRi_ClimaCfgHvacReForRowSecForActrTRi_read/1 -> Terminator_23/1
  ClimaCfgReqVersionReplace_ClimaCfgReqVersionReplace_read/1 -> Terminator_24/1
  ClimaClsDwn_ClimaClsDwn_read/1 -> Terminator_25/1
  ClimaCtrlActvNgcc_ClimaCtrlActv_read/1 -> Terminator_26/1
  FlowRednAtPwrLvlLo_FlowRednAtPwrLvlLo_read/1 -> Terminator_27/1
  HmiCmptmtAirDistbnFrntForClima_HmiCmptmtAirDistbnFrntForClima_read/1 -> Terminator_28/1
  HmiCmptmtAirDistbnFrntForClima_HmiCmptmtAirDistbnFrntRiForClima_read/1 -> Terminator_29/1
  HmiCmptmtAirDistbnFrntForClima_HmiCmptmtAirDistbnReForClima_read/1 -> Terminator_30/1
  HmiCmptmtAirDistbnFrntForClima_HmiCmptmtAirDistbnReRiForClima_read/1 -> Terminator_31/1
  HmiHvacFanLvlReForClima_HmiHvacFanLvlReForClima_read/1 -> Terminator_32/1
  HmiHvacFanLvlReForClima_HmiHvacFanLvlReRiForClima_read/1 -> Terminator_33/1
  HvacAirTReqAtRowSecLe_HvacAirTReqAtRowSecLe_read/1 -> Terminator_34/1
  HvacAirTReqAtRowSecRi_HvacAirTReqAtRowSecRi_read/1 -> Terminator_35/1
  HvacCtrlrReqReForRowSecForActrTLe_HvacCtrlrReqReForRowSecForActrTLe_read/1 -> Terminator_36/1
  HvacCtrlrReqReForRowSecForActrTRi_HvacCtrlrReqReForRowSecForActrTRi_read/1 -> Terminator_37/1
  HvacFanFrntLimForPwrAllwd_HvacFanFrntLimForPwrAllwd_read/1 -> Terminator_38/1
  HvacFanSpdReNgcc_HvacFanSpdReNgcc_read/1 -> Terminator_39/1
  HvacFlowReReqForRowThrdAtFlr_HvacFlowReReqForRowThrdAtFlr_read/1 -> Terminator_40/1
  HvacFlowReReqForRowThrdAtVent_HvacFlowReReqForRowThrdAtVent_read/1 -> Terminator_41/1
  HvacLpmUFbRe_HvacLpmUFbRe_read/1 -> Terminator_42/1
  HvacTarFlowTotRe_HvacTarFlowTotRe_read/1 -> Terminator_43/1
  LdAdVal_CurrAdVal_read/1 -> Terminator_44/1
  LdAdVal_ValidAdVal_read/1 -> Terminator_45/1
  MemReadForHvacActrPosnReqFrnt_MemReadForHvacActrPosnReqFrnt_read/1 -> Terminator_46/1
  MemReqStsForHvac_MemReqStsForHvac_read/1 -> Terminator_47/1
  MemWrForHvacActrPosnReqFrnt_MemWrForHvacActrPosnReqFrnt_read/1 -> Terminator_48/1
  PrkgClimaOnReq_PrkgClimaOnReq_read/1 -> Terminator_49/1
  PrkgClimaOnReqRe_PrkgClimaOnReqRe_read/1 -> Terminator_50/1
  PwrAllwdForHvacFanRe_PwrAllwdForHvacFanRe_read/1 -> Terminator_51/1
  ReBlwWrkLmt_ReBlwWrkLmt_read/1 -> Terminator_52/1
  VehModMngtGlbSafe1_Ngcc_VehModMngtGlbSafe1_read/1 -> Terminator_53/1
  VehSpdLgtForClima_VehSpdLgtForClima_read/1 -> Terminator_54/1
  Ground_1/1 -> ActrReqForModDistbnRe_ActrReqForModDistbnRe_write/1
  Ground_2/1 -> HvacAirMFlowEstimdForCtrlrRe_HvacAirMFlowEstimdForCtrlrRe_write/1
  Ground_3/1 -> HvacAirMFlowEstimdInHeatElmReNgcc_HvacAirMFlowEstimdInHeatElmReNgcc_write/1
  Ground_4/1 -> HvacAirMFlowMaxEstimdRe_HvacAirMFlowMaxEstimdRe_write/1
  Ground_5/1 -> HvacAirMFlowReEstimd_Ngcc_HvacAirMFlowReEstimd_write/1
  Ground_6/1 -> HvacCtrlReqForActrModRe_HvacCtrlReqForActrModRe_write/1
  Ground_7/1 -> HvacFanDutyReqRe_HvacFanDutyReqRe_write/1
  Ground_8/1 -> HvacFanLimForPwrAllwdRe_HvacFanLimForPwrAllwdRe_write/1
  Ground_9/1 -> HvacFanLvlFbReNgcc_HvacFanLvlFbReNgcc_write/1
  Ground_10/1 -> HvacFanReqRe_HvacFanReqRe_write/1
  Ground_11/1 -> HvacFanVoltReqRe_HvacFanVoltReqRe_write/1
  Ground_12/1 -> HvacFlowRednEnadRe_HvacFlowRednEnadRe_write/1
  Ground_13/1 -> HvacFlowReForRowSecAtFlrLeRe_HvacFlowReForRowSecAtFlrLeRe_write/1
  Ground_14/1 -> HvacFlowReForRowSecAtFlrRiRe_HvacFlowReForRowSecAtFlrRiRe_write/1
  Ground_15/1 -> HvacFlowReForRowSecAtVentSideTopLe_HvacFlowReForRowSecAtVentSideTopLe_write/1
  Ground_16/1 -> HvacFlowReForRowSecAtVentSideTopRi_HvacFlowReForRowSecAtVentSideTopRi_write/1
  Ground_17/1 -> HvacFlowReForRowThrdAtFlrLe_HvacFlowReForRowThrdAtFlrLe_write/1
  Ground_18/1 -> HvacFlowReForRowThrdAtFlrRi_HvacFlowReForRowThrdAtFlrRi_write/1
  Ground_19/1 -> HvacFlowReForRowThrdAtVentSideTopLe_HvacFlowReForRowThrdAtVentSideTopLe_write/1
  Ground_20/1 -> HvacFlowReForRowThrdAtVentSideTopRi_HvacFlowReForRowThrdAtVentSideTopRi_write/1
  Ground_21/1 -> ManModeForRowThrdForModelDial_ManModeForRowThrdForModelDial_write/1
  Ground_22/1 -> PwrCnsDesForHvacFanRe_PwrCnsDesForHvacFanRe_write/1
  Ground_23/1 -> PwrCnsForHvacFanReNgcc_PwrCnsForHvacFanRe_write/1
  Ground_24/1 -> UBoostReqByClimaRe_UBoostReqByClimaRe_write/1
